simulink model "ofdm_txrx_supermimo_coded"  (Simulink 7.7) (continued)
== system ofdm_txrx_supermimo_coded/EDK Processor == (continued)
      | sm_TxRx_AutoReply_Match3_dout = xl_force(sm_TxRx_AutoReply_Match3, xlUnsigned, 0);
      | 
      | % sm_TxRx_AutoReply_Match2_dout = xfix({xlUnsigned, 32, 0}, 0);
      | sm_TxRx_AutoReply_Match2_dout = xl_force(sm_TxRx_AutoReply_Match2, xlUnsigned, 0);
      | 
      | % sm_TxRx_AutoReply_Action5_dout = xfix({xlUnsigned, 32, 0}, 0);
      | sm_TxRx_AutoReply_Action5_dout = xl_force(sm_TxRx_AutoReply_Action5, xlUnsigned, 0);
      | 
      | % sm_TxRx_AutoReply_Action4_dout = xfix({xlUnsigned, 32, 0}, 0);
      | sm_TxRx_AutoReply_Action4_dout = xl_force(sm_TxRx_AutoReply_Action4, xlUnsigned, 0);
      | 
      | % sm_TxRx_AutoReply_Match1_dout = xfix({xlUnsigned, 32, 0}, 0);
      | sm_TxRx_AutoReply_Match1_dout = xl_force(sm_TxRx_AutoReply_Match1, xlUnsigned, 0);
      | 
      | % sm_TxRx_AutoReply_Match0_dout = xfix({xlUnsigned, 32, 0}, 0);
      | sm_TxRx_AutoReply_Match0_dout = xl_force(sm_TxRx_AutoReply_Match0, xlUnsigned, 0);
      | 
      | 
      | % 'From FIFO' blocks
      | % 'To FIFO' blocks
      | % 'Shared Memory' blocks
      | % TxModulation_bus = xfix({xlUnsigned, 32, 0}, 0);
      | sm_TxModulation_bus = xl_force(sm_TxModulation, xlUnsigned, 0);
      | 
      | % TxHeaderTranslate_bus = xfix({xlUnsigned, 32, 0}, 0);
      | sm_TxHeaderTranslate_bus = xl_force(sm_TxHeaderTranslate, xlUnsigned, 0);
      | 
      | % ChannelEstimates_bus = xfix({xlUnsigned, 32, 0}, 0);
      | sm_ChannelEstimates_bus = xl_force(sm_ChannelEstimates, xlUnsigned, 0);
      | 
      | % RxModulation_bus = xfix({xlUnsigned, 32, 0}, 0);
      | sm_RxModulation_bus = xl_force(sm_RxModulation, xlUnsigned, 0);
      | 
      | % EVM_perSym_bus = xfix({xlUnsigned, 32, 0}, 0);
      | sm_EVM_perSym_bus = xl_force(sm_EVM_perSym, xlUnsigned, 0);
      | 
      | % EVM_perSC_bus = xfix({xlUnsigned, 32, 0}, 0);
      | sm_EVM_perSC_bus = xl_force(sm_EVM_perSC, xlUnsigned, 0);
      | 
      | % PktBufFreqOffsets_bus = xfix({xlUnsigned, 32, 0}, 0);
      | sm_PktBufFreqOffsets_bus = xl_force(sm_PktBufFreqOffsets, xlUnsigned, 0);
      | 
      | 
      | % 'dout' ports of 'From Register' blocks
      | 
      | % registered register mux output
      | persistent reg_bank_out_reg; reg_bank_out_reg = xl_state(0, {xlUnsigned, 32, 0});
      | reg_bank_out = reg_bank_out_reg;
      | 
      | if linearAddr == 41
      |     reg_bank_out_reg = sm_midPacketRSSI_bus;
      | elseif linearAddr == 42
      |     reg_bank_out_reg = sm_Tx_PktRunning_bus;
      | elseif linearAddr == 43
      |     reg_bank_out_reg = sm_Rx_pktDetEventCount_bus;
      | elseif linearAddr == 44
      |     reg_bank_out_reg = sm_Rx_coarseCFOest_bus;
      | elseif linearAddr == 45
      |     reg_bank_out_reg = sm_Rx_pilotCFOest_bus;
      | elseif linearAddr == 46
      |     reg_bank_out_reg = sm_Rx_pktDone_interruptStatus_bus;
      | elseif linearAddr == 47
      |     reg_bank_out_reg = sm_Rx_BER_TotalBits_bus;
      | elseif linearAddr == 48
      |     reg_bank_out_reg = sm_Rx_BER_Errors_bus;
      | elseif linearAddr == 49
      |     reg_bank_out_reg = sm_Rx_Gains_bus;
      | elseif linearAddr == 50
      |     reg_bank_out_reg = sm_pktDet_status_bus;
      | elseif linearAddr == 0
      |     reg_bank_out_reg = sm_FEC_Config_dout;
      | elseif linearAddr == 1
      |     reg_bank_out_reg = sm_Tx_OFDM_SymCounts_dout;
      | elseif linearAddr == 2
      |     reg_bank_out_reg = sm_Rx_OFDM_SymbolCounts_dout;
      | elseif linearAddr == 3
      |     reg_bank_out_reg = sm_TxRx_Pilots_Values_dout;
      | elseif linearAddr == 4
      |     reg_bank_out_reg = sm_TxRx_Pilots_Index_dout;
      | elseif linearAddr == 5
      |     reg_bank_out_reg = sm_TxRx_Interrupt_PktBuf_Ctrl_dout;
      | elseif linearAddr == 6
      |     reg_bank_out_reg = sm_TxRx_FFT_Scaling_dout;
      | elseif linearAddr == 7
      |     reg_bank_out_reg = sm_Tx_Start_Reset_Control_dout;
      | elseif linearAddr == 8
      |     reg_bank_out_reg = sm_Tx_ControlBits_dout;
      | elseif linearAddr == 9
      |     reg_bank_out_reg = sm_Tx_Delays_dout;
      | elseif linearAddr == 10
      |     reg_bank_out_reg = sm_Tx_Scaling_dout;
      | elseif linearAddr == 11
      |     reg_bank_out_reg = sm_pktDet_thresholds_dout;
      | elseif linearAddr == 12
      |     reg_bank_out_reg = sm_pktDet_durations_dout;
      | elseif linearAddr == 13
      |     reg_bank_out_reg = sm_pktDet_autoCorrParams_dout;
      | elseif linearAddr == 14
      |     reg_bank_out_reg = sm_pktDet_controlBits_dout;
      | elseif linearAddr == 15
      |     reg_bank_out_reg = sm_Rx_AF_TxScaling_dout;
      | elseif linearAddr == 16
      |     reg_bank_out_reg = sm_Rx_AF_Blanking_dout;
      | elseif linearAddr == 17
      |     reg_bank_out_reg = sm_Rx_PilotCalcParams_dout;
      | elseif linearAddr == 18
      |     reg_bank_out_reg = sm_Rx_Constellation_Scaling_dout;
      | elseif linearAddr == 19
      |     reg_bank_out_reg = sm_Rx_PktDet_Delay_dout;
      | elseif linearAddr == 20
      |     reg_bank_out_reg = sm_Rx_ChanEst_MinMag_dout;
      | elseif linearAddr == 21
      |     reg_bank_out_reg = sm_Rx_pktByteNums_dout;
      | elseif linearAddr == 22
      |     reg_bank_out_reg = sm_Rx_FixedPktLen_dout;
      | elseif linearAddr == 23
      |     reg_bank_out_reg = sm_Rx_PktDet_LongCorr_Thresholds_dout;
      | elseif linearAddr == 24
      |     reg_bank_out_reg = sm_Rx_PktDet_LongCorr_Params_dout;
      | elseif linearAddr == 25
      |     reg_bank_out_reg = sm_Rx_coarseCFO_correction_dout;
      | elseif linearAddr == 26
      |     reg_bank_out_reg = sm_Rx_PreCFO_PilotCalcCorrection_dout;
      | elseif linearAddr == 27
      |     reg_bank_out_reg = sm_Rx_PreCFO_Options_dout;
      | elseif linearAddr == 28
      |     reg_bank_out_reg = sm_Rx_ControlBits_dout;
      | elseif linearAddr == 29
      |     reg_bank_out_reg = sm_TxRx_AutoReply_Action3_dout;
      | elseif linearAddr == 30
      |     reg_bank_out_reg = sm_TxRx_AutoReply_Action2_dout;
      | elseif linearAddr == 31
      |     reg_bank_out_reg = sm_TxRx_AutoReply_Action1_dout;
      | elseif linearAddr == 32
      |     reg_bank_out_reg = sm_TxRx_AutoReply_Action0_dout;
      | elseif linearAddr == 33
      |     reg_bank_out_reg = sm_TxRx_AutoReply_Match5_dout;
      | elseif linearAddr == 34
      |     reg_bank_out_reg = sm_TxRx_AutoReply_Match4_dout;
      | elseif linearAddr == 35
      |     reg_bank_out_reg = sm_TxRx_AutoReply_Match3_dout;
      | elseif linearAddr == 36
      |     reg_bank_out_reg = sm_TxRx_AutoReply_Match2_dout;
      | elseif linearAddr == 37
      |     reg_bank_out_reg = sm_TxRx_AutoReply_Action5_dout;
      | elseif linearAddr == 38
      |     reg_bank_out_reg = sm_TxRx_AutoReply_Action4_dout;
      | elseif linearAddr == 39
      |     reg_bank_out_reg = sm_TxRx_AutoReply_Match1_dout;
      | elseif linearAddr == 40
      |     reg_bank_out_reg = sm_TxRx_AutoReply_Match0_dout;
      | 
      | end
      | 
      | 
      | % 'From FIFO' and 'To FIFO' blocks
      | 
      | 
      | 
      | 
      | 
      | opCode = xl_concat(addrAck, RNWReg, bankAddr, linearAddr);
      | 
      | % 'Shared Memory' blocks
      | 
      | sm_TxModulation_sel_value = xl_concat(xl_slice(linearAddr, ...
      |                                         xl_nbits(linearAddr) - 1, ...
      |                                         8) ...
      |                                     );
      | if sm_TxModulation_sel_value == xfix({xlUnsigned, ...
      |                                         xl_nbits(linearAddr) - 8, ...
      |                                         0}, ...
      |                                         4);
      |     sm_TxModulation_sel = true;
      | else
      |     sm_TxModulation_sel = false;
      | end
      | sm_TxHeaderTranslate_sel_value = xl_concat(xl_slice(linearAddr, ...
      |                                         xl_nbits(linearAddr) - 1, ...
      |                                         10) ...
      |                                     );
      | if sm_TxHeaderTranslate_sel_value == xfix({xlUnsigned, ...
      |                                         xl_nbits(linearAddr) - 10, ...
      |                                         0}, ...
      |                                         0);
      |     sm_TxHeaderTranslate_sel = true;
      | else
      |     sm_TxHeaderTranslate_sel = false;
      | end
      | sm_ChannelEstimates_sel_value = xl_concat(xl_slice(linearAddr, ...
      |                                         xl_nbits(linearAddr) - 1, ...
      |                                         8) ...
      |                                     );
      | if sm_ChannelEstimates_sel_value == xfix({xlUnsigned, ...
      |                                         xl_nbits(linearAddr) - 8, ...
      |                                         0}, ...
      |                                         5);
      |     sm_ChannelEstimates_sel = true;
      | else
      |     sm_ChannelEstimates_sel = false;
      | end
      | sm_RxModulation_sel_value = xl_concat(xl_slice(linearAddr, ...
      |                                         xl_nbits(linearAddr) - 1, ...
      |                                         8) ...
      |                                     );
      | if sm_RxModulation_sel_value == xfix({xlUnsigned, ...
      |                                         xl_nbits(linearAddr) - 8, ...
      |                                         0}, ...
      |                                         6);
      |     sm_RxModulation_sel = true;
      | else
      |     sm_RxModulation_sel = false;
      | end
      | sm_EVM_perSym_sel_value = xl_concat(xl_slice(linearAddr, ...
      |                                         xl_nbits(linearAddr) - 1, ...
      |                                         8) ...
      |                                     );
      | if sm_EVM_perSym_sel_value == xfix({xlUnsigned, ...
      |                                         xl_nbits(linearAddr) - 8, ...
      |                                         0}, ...
      |                                         7);
      |     sm_EVM_perSym_sel = true;
      | else
      |     sm_EVM_perSym_sel = false;
      | end
      | sm_EVM_perSC_sel_value = xl_concat(xl_slice(linearAddr, ...
      |                                         xl_nbits(linearAddr) - 1, ...
      |                                         6) ...
      |                                     );
      | if sm_EVM_perSC_sel_value == xfix({xlUnsigned, ...
      |                                         xl_nbits(linearAddr) - 6, ...
      |                                         0}, ...
      |                                         32);
      |     sm_EVM_perSC_sel = true;
      | else
      |     sm_EVM_perSC_sel = false;
      | end
      | sm_PktBufFreqOffsets_sel_value = xl_concat(xl_slice(linearAddr, ...
      |                                         xl_nbits(linearAddr) - 1, ...
      |                                         5) ...
      |                                     );
      | if sm_PktBufFreqOffsets_sel_value == xfix({xlUnsigned, ...
      |                                         xl_nbits(linearAddr) - 5, ...
      |                                         0}, ...
      |                                         66);
      |     sm_PktBufFreqOffsets_sel = true;
      | else
      |     sm_PktBufFreqOffsets_sel = false;
      | end
      | 
      | 
      | % registered Shared Memory mux output
      | persistent ram_bank_out_reg; ram_bank_out_reg = xl_state(0, {xlUnsigned, 32, 0});
      | ram_bank_out = ram_bank_out_reg;
      | if sm_TxModulation_sel
      |     ram_bank_out_reg = sm_TxModulation_bus;
      | elseif sm_TxHeaderTranslate_sel
      |     ram_bank_out_reg = sm_TxHeaderTranslate_bus;
      | elseif sm_ChannelEstimates_sel
      |     ram_bank_out_reg = sm_ChannelEstimates_bus;
      | elseif sm_RxModulation_sel
      |     ram_bank_out_reg = sm_RxModulation_bus;
      | elseif sm_EVM_perSym_sel
      |     ram_bank_out_reg = sm_EVM_perSym_bus;
      | elseif sm_EVM_perSC_sel
      |     ram_bank_out_reg = sm_EVM_perSC_bus;
      | elseif sm_PktBufFreqOffsets_sel
      |     ram_bank_out_reg = sm_PktBufFreqOffsets_bus;
      | end
      | 
      | % 'din' ports of 'Shared Memory' blocks
      | sm_TxModulation_din = xl_force(xl_slice(wrDBus, 4 - 1, 0), ...
      |                                  xlUnsigned, ...
      |                                  0);
      | sm_TxHeaderTranslate_din = xl_force(xl_slice(wrDBus, 10 - 1, 0), ...
      |                                  xlUnsigned, ...
      |                                  0);
      | sm_ChannelEstimates_din = xl_force(xl_slice(wrDBus, 32 - 1, 0), ...
      |                                  xlUnsigned, ...
      |                                  0);
      | sm_RxModulation_din = xl_force(xl_slice(wrDBus, 4 - 1, 0), ...
      |                                  xlUnsigned, ...
      |                                  0);
      | sm_EVM_perSym_din = xl_force(xl_slice(wrDBus, 32 - 1, 0), ...
      |                                  xlSigned, ...
      |                                  14);
      | sm_EVM_perSC_din = xl_force(xl_slice(wrDBus, 32 - 1, 0), ...
      |                                  xlSigned, ...
      |                                  22);
      | sm_PktBufFreqOffsets_din = xl_force(xl_slice(wrDBus, 32 - 1, 0), ...
      |                                  xlUnsigned, ...
      |                                  32);
      | 
      | 
      | % 'we' ports of 'Shared Memory' blocks
      | persistent sm_TxModulation_we_reg; sm_TxModulation_we_reg = xl_state(false, {xlBoolean});
      | sm_TxModulation_we = sm_TxModulation_we_reg;
      | opCode_sm_TxModulation = xl_concat(addrAck, ...
      |                                      RNWReg, ...
      |                                      bankAddr, ...
      |                                      xl_slice(linearAddr, ...
      |                                               xl_nbits(linearAddr) - 1, ...
      |                                               8) ...
      |                                     );
      | if opCode_sm_TxModulation == xl_concat(xfix({xlUnsigned, 4, 0}, 8), ...
      |                                          xfix({xlUnsigned, ...
      |                                                xl_nbits(linearAddr) - 8, ...
      |                                                0}, ...
      |                                                4) ...
      |                                         );
      |     sm_TxModulation_we_reg = true;
      | else
      |     sm_TxModulation_we_reg = false;
      | end
      | persistent sm_TxHeaderTranslate_we_reg; sm_TxHeaderTranslate_we_reg = xl_state(false, {xlBoolean});
      | sm_TxHeaderTranslate_we = sm_TxHeaderTranslate_we_reg;
      | opCode_sm_TxHeaderTranslate = xl_concat(addrAck, ...
      |                                      RNWReg, ...
      |                                      bankAddr, ...
      |                                      xl_slice(linearAddr, ...
      |                                               xl_nbits(linearAddr) - 1, ...
      |                                               10) ...
      |                                     );
      | if opCode_sm_TxHeaderTranslate == xl_concat(xfix({xlUnsigned, 4, 0}, 8), ...
      |                                          xfix({xlUnsigned, ...
      |                                                xl_nbits(linearAddr) - 10, ...
      |                                                0}, ...
      |                                                0) ...
      |                                         );
      |     sm_TxHeaderTranslate_we_reg = true;
      | else
      |     sm_TxHeaderTranslate_we_reg = false;
      | end
      | persistent sm_ChannelEstimates_we_reg; sm_ChannelEstimates_we_reg = xl_state(false, {xlBoolean});
      | sm_ChannelEstimates_we = sm_ChannelEstimates_we_reg;
      | opCode_sm_ChannelEstimates = xl_concat(addrAck, ...
      |                                      RNWReg, ...
      |                                      bankAddr, ...
      |                                      xl_slice(linearAddr, ...
      |                                               xl_nbits(linearAddr) - 1, ...
      |                                               8) ...
      |                                     );
      | if opCode_sm_ChannelEstimates == xl_concat(xfix({xlUnsigned, 4, 0}, 8), ...
      |                                          xfix({xlUnsigned, ...
      |                                                xl_nbits(linearAddr) - 8, ...
      |                                                0}, ...
      |                                                5) ...
      |                                         );
      |     sm_ChannelEstimates_we_reg = true;
      | else
      |     sm_ChannelEstimates_we_reg = false;
      | end
      | persistent sm_RxModulation_we_reg; sm_RxModulation_we_reg = xl_state(false, {xlBoolean});
      | sm_RxModulation_we = sm_RxModulation_we_reg;
      | opCode_sm_RxModulation = xl_concat(addrAck, ...
      |                                      RNWReg, ...
      |                                      bankAddr, ...
      |                                      xl_slice(linearAddr, ...
      |                                               xl_nbits(linearAddr) - 1, ...
      |                                               8) ...
      |                                     );
      | if opCode_sm_RxModulation == xl_concat(xfix({xlUnsigned, 4, 0}, 8), ...
      |                                          xfix({xlUnsigned, ...
      |                                                xl_nbits(linearAddr) - 8, ...
      |                                                0}, ...
      |                                                6) ...
      |                                         );
      |     sm_RxModulation_we_reg = true;
      | else
      |     sm_RxModulation_we_reg = false;
      | end
      | persistent sm_EVM_perSym_we_reg; sm_EVM_perSym_we_reg = xl_state(false, {xlBoolean});
      | sm_EVM_perSym_we = sm_EVM_perSym_we_reg;
      | opCode_sm_EVM_perSym = xl_concat(addrAck, ...
      |                                      RNWReg, ...
      |                                      bankAddr, ...
      |                                      xl_slice(linearAddr, ...
      |                                               xl_nbits(linearAddr) - 1, ...
      |                                               8) ...
      |                                     );
      | if opCode_sm_EVM_perSym == xl_concat(xfix({xlUnsigned, 4, 0}, 8), ...
      |                                          xfix({xlUnsigned, ...
      |                                                xl_nbits(linearAddr) - 8, ...
      |                                                0}, ...
      |                                                7) ...
      |                                         );
      |     sm_EVM_perSym_we_reg = true;
      | else
      |     sm_EVM_perSym_we_reg = false;
      | end
      | persistent sm_EVM_perSC_we_reg; sm_EVM_perSC_we_reg = xl_state(false, {xlBoolean});
      | sm_EVM_perSC_we = sm_EVM_perSC_we_reg;
      | opCode_sm_EVM_perSC = xl_concat(addrAck, ...
      |                                      RNWReg, ...
      |                                      bankAddr, ...
      |                                      xl_slice(linearAddr, ...
      |                                               xl_nbits(linearAddr) - 1, ...
      |                                               6) ...
      |                                     );
      | if opCode_sm_EVM_perSC == xl_concat(xfix({xlUnsigned, 4, 0}, 8), ...
      |                                          xfix({xlUnsigned, ...
      |                                                xl_nbits(linearAddr) - 6, ...
      |                                                0}, ...
      |                                                32) ...
      |                                         );
      |     sm_EVM_perSC_we_reg = true;
      | else
      |     sm_EVM_perSC_we_reg = false;
      | end
      | persistent sm_PktBufFreqOffsets_we_reg; sm_PktBufFreqOffsets_we_reg = xl_state(false, {xlBoolean});
      | sm_PktBufFreqOffsets_we = sm_PktBufFreqOffsets_we_reg;
      | opCode_sm_PktBufFreqOffsets = xl_concat(addrAck, ...
      |                                      RNWReg, ...
      |                                      bankAddr, ...
      |                                      xl_slice(linearAddr, ...
      |                                               xl_nbits(linearAddr) - 1, ...
      |                                               5) ...
      |                                     );
      | if opCode_sm_PktBufFreqOffsets == xl_concat(xfix({xlUnsigned, 4, 0}, 8), ...
      |                                          xfix({xlUnsigned, ...
      |                                                xl_nbits(linearAddr) - 5, ...
      |                                                0}, ...
      |                                                66) ...
      |                                         );
      |     sm_PktBufFreqOffsets_we_reg = true;
      | else
      |     sm_PktBufFreqOffsets_we_reg = false;
      | end
      | 
      | 
      | % 'addr' ports of 'Shared Memory' blocks
      | persistent sm_TxModulation_addr_reg; 
      | sm_TxModulation_addr_reg = xl_state(0, {xlUnsigned, 8, 0});
      | sm_TxModulation_addr = sm_TxModulation_addr_reg;
      | if addrAck == 1
      |     sm_TxModulation_addr_reg = xl_slice(linearAddr, 8, 0);
      | else
      |     sm_TxModulation_addr_reg = sm_TxModulation_addr_reg;
      | end
      | persistent sm_TxHeaderTranslate_addr_reg; 
      | sm_TxHeaderTranslate_addr_reg = xl_state(0, {xlUnsigned, 10, 0});
      | sm_TxHeaderTranslate_addr = sm_TxHeaderTranslate_addr_reg;
      | if addrAck == 1
      |     sm_TxHeaderTranslate_addr_reg = xl_slice(linearAddr, 10, 0);
      | else
      |     sm_TxHeaderTranslate_addr_reg = sm_TxHeaderTranslate_addr_reg;
      | end
      | persistent sm_ChannelEstimates_addr_reg; 
      | sm_ChannelEstimates_addr_reg = xl_state(0, {xlUnsigned, 8, 0});
      | sm_ChannelEstimates_addr = sm_ChannelEstimates_addr_reg;
      | if addrAck == 1
      |     sm_ChannelEstimates_addr_reg = xl_slice(linearAddr, 8, 0);
      | else
      |     sm_ChannelEstimates_addr_reg = sm_ChannelEstimates_addr_reg;
      | end
      | persistent sm_RxModulation_addr_reg; 
      | sm_RxModulation_addr_reg = xl_state(0, {xlUnsigned, 8, 0});
      | sm_RxModulation_addr = sm_RxModulation_addr_reg;
      | if addrAck == 1
      |     sm_RxModulation_addr_reg = xl_slice(linearAddr, 8, 0);
      | else
      |     sm_RxModulation_addr_reg = sm_RxModulation_addr_reg;
      | end
      | persistent sm_EVM_perSym_addr_reg; 
      | sm_EVM_perSym_addr_reg = xl_state(0, {xlUnsigned, 8, 0});
      | sm_EVM_perSym_addr = sm_EVM_perSym_addr_reg;
      | if addrAck == 1
      |     sm_EVM_perSym_addr_reg = xl_slice(linearAddr, 8, 0);
      | else
      |     sm_EVM_perSym_addr_reg = sm_EVM_perSym_addr_reg;
      | end
      | persistent sm_EVM_perSC_addr_reg; 
      | sm_EVM_perSC_addr_reg = xl_state(0, {xlUnsigned, 6, 0});
      | sm_EVM_perSC_addr = sm_EVM_perSC_addr_reg;
      | if addrAck == 1
      |     sm_EVM_perSC_addr_reg = xl_slice(linearAddr, 6, 0);
      | else
      |     sm_EVM_perSC_addr_reg = sm_EVM_perSC_addr_reg;
      | end
      | persistent sm_PktBufFreqOffsets_addr_reg; 
      | sm_PktBufFreqOffsets_addr_reg = xl_state(0, {xlUnsigned, 5, 0});
      | sm_PktBufFreqOffsets_addr = sm_PktBufFreqOffsets_addr_reg;
      | if addrAck == 1
      |     sm_PktBufFreqOffsets_addr_reg = xl_slice(linearAddr, 5, 0);
      | else
      |     sm_PktBufFreqOffsets_addr_reg = sm_PktBufFreqOffsets_addr_reg;
      | end
      | 
      | 
      | % 're' ports of 'From FIFO' blocks
      | 
      | 
      | % 'en' ports of 'To Register' blocks
      | if opCode == xl_concat(xfix({xlUnsigned, 4, 0}, 10), ...
      |                        xfix({xlUnsigned, xl_nbits(linearAddr), 0}, 0))
      |     sm_FEC_Config_en = true;
      | else
      |     sm_FEC_Config_en = false;
      | end
      | if opCode == xl_concat(xfix({xlUnsigned, 4, 0}, 10), ...
      |                        xfix({xlUnsigned, xl_nbits(linearAddr), 0}, 1))
      |     sm_Tx_OFDM_SymCounts_en = true;
      | else
      |     sm_Tx_OFDM_SymCounts_en = false;
      | end
      | if opCode == xl_concat(xfix({xlUnsigned, 4, 0}, 10), ...
      |                        xfix({xlUnsigned, xl_nbits(linearAddr), 0}, 2))
      |     sm_Rx_OFDM_SymbolCounts_en = true;
      | else
      |     sm_Rx_OFDM_SymbolCounts_en = false;
      | end
      | if opCode == xl_concat(xfix({xlUnsigned, 4, 0}, 10), ...
      |                        xfix({xlUnsigned, xl_nbits(linearAddr), 0}, 3))
      |     sm_TxRx_Pilots_Values_en = true;
      | else
      |     sm_TxRx_Pilots_Values_en = false;
      | end
      | if opCode == xl_concat(xfix({xlUnsigned, 4, 0}, 10), ...
      |                        xfix({xlUnsigned, xl_nbits(linearAddr), 0}, 4))
      |     sm_TxRx_Pilots_Index_en = true;
      | else
      |     sm_TxRx_Pilots_Index_en = false;
      | end
      | if opCode == xl_concat(xfix({xlUnsigned, 4, 0}, 10), ...
      |                        xfix({xlUnsigned, xl_nbits(linearAddr), 0}, 5))
      |     sm_TxRx_Interrupt_PktBuf_Ctrl_en = true;
      | else
      |     sm_TxRx_Interrupt_PktBuf_Ctrl_en = false;
      | end
      | if opCode == xl_concat(xfix({xlUnsigned, 4, 0}, 10), ...
      |                        xfix({xlUnsigned, xl_nbits(linearAddr), 0}, 6))
      |     sm_TxRx_FFT_Scaling_en = true;
      | else
      |     sm_TxRx_FFT_Scaling_en = false;
      | end
      | if opCode == xl_concat(xfix({xlUnsigned, 4, 0}, 10), ...
      |                        xfix({xlUnsigned, xl_nbits(linearAddr), 0}, 7))
      |     sm_Tx_Start_Reset_Control_en = true;
      | else
      |     sm_Tx_Start_Reset_Control_en = false;
      | end
      | if opCode == xl_concat(xfix({xlUnsigned, 4, 0}, 10), ...
      |                        xfix({xlUnsigned, xl_nbits(linearAddr), 0}, 8))
      |     sm_Tx_ControlBits_en = true;
      | else
      |     sm_Tx_ControlBits_en = false;
      | end
      | if opCode == xl_concat(xfix({xlUnsigned, 4, 0}, 10), ...
      |                        xfix({xlUnsigned, xl_nbits(linearAddr), 0}, 9))
      |     sm_Tx_Delays_en = true;
      | else
      |     sm_Tx_Delays_en = false;
      | end
      | if opCode == xl_concat(xfix({xlUnsigned, 4, 0}, 10), ...
      |                        xfix({xlUnsigned, xl_nbits(linearAddr), 0}, 10))
      |     sm_Tx_Scaling_en = true;
      | else
      |     sm_Tx_Scaling_en = false;
      | end
      | if opCode == xl_concat(xfix({xlUnsigned, 4, 0}, 10), ...
      |                        xfix({xlUnsigned, xl_nbits(linearAddr), 0}, 11))
      |     sm_pktDet_thresholds_en = true;
      | else
      |     sm_pktDet_thresholds_en = false;
      | end
      | if opCode == xl_concat(xfix({xlUnsigned, 4, 0}, 10), ...
      |                        xfix({xlUnsigned, xl_nbits(linearAddr), 0}, 12))
      |     sm_pktDet_durations_en = true;
      | else
      |     sm_pktDet_durations_en = false;
      | end
      | if opCode == xl_concat(xfix({xlUnsigned, 4, 0}, 10), ...
      |                        xfix({xlUnsigned, xl_nbits(linearAddr), 0}, 13))
      |     sm_pktDet_autoCorrParams_en = true;
      | else
      |     sm_pktDet_autoCorrParams_en = false;
      | end
      | if opCode == xl_concat(xfix({xlUnsigned, 4, 0}, 10), ...
      |                        xfix({xlUnsigned, xl_nbits(linearAddr), 0}, 14))
      |     sm_pktDet_controlBits_en = true;
      | else
      |     sm_pktDet_controlBits_en = false;
      | end
      | if opCode == xl_concat(xfix({xlUnsigned, 4, 0}, 10), ...
      |                        xfix({xlUnsigned, xl_nbits(linearAddr), 0}, 15))
      |     sm_Rx_AF_TxScaling_en = true;
      | else
      |     sm_Rx_AF_TxScaling_en = false;
      | end
      | if opCode == xl_concat(xfix({xlUnsigned, 4, 0}, 10), ...
      |                        xfix({xlUnsigned, xl_nbits(linearAddr), 0}, 16))
      |     sm_Rx_AF_Blanking_en = true;
      | else
      |     sm_Rx_AF_Blanking_en = false;
      | end
      | if opCode == xl_concat(xfix({xlUnsigned, 4, 0}, 10), ...
      |                        xfix({xlUnsigned, xl_nbits(linearAddr), 0}, 17))
      |     sm_Rx_PilotCalcParams_en = true;
      | else
      |     sm_Rx_PilotCalcParams_en = false;
      | end
      | if opCode == xl_concat(xfix({xlUnsigned, 4, 0}, 10), ...
      |                        xfix({xlUnsigned, xl_nbits(linearAddr), 0}, 18))
      |     sm_Rx_Constellation_Scaling_en = true;
      | else
      |     sm_Rx_Constellation_Scaling_en = false;
      | end
      | if opCode == xl_concat(xfix({xlUnsigned, 4, 0}, 10), ...
      |                        xfix({xlUnsigned, xl_nbits(linearAddr), 0}, 19))
      |     sm_Rx_PktDet_Delay_en = true;
      | else
      |     sm_Rx_PktDet_Delay_en = false;
      | end
      | if opCode == xl_concat(xfix({xlUnsigned, 4, 0}, 10), ...
      |                        xfix({xlUnsigned, xl_nbits(linearAddr), 0}, 20))
      |     sm_Rx_ChanEst_MinMag_en = true;
      | else
      |     sm_Rx_ChanEst_MinMag_en = false;
      | end
      | if opCode == xl_concat(xfix({xlUnsigned, 4, 0}, 10), ...
      |                        xfix({xlUnsigned, xl_nbits(linearAddr), 0}, 21))
      |     sm_Rx_pktByteNums_en = true;
      | else
      |     sm_Rx_pktByteNums_en = false;
      | end
      | if opCode == xl_concat(xfix({xlUnsigned, 4, 0}, 10), ...
      |                        xfix({xlUnsigned, xl_nbits(linearAddr), 0}, 22))
      |     sm_Rx_FixedPktLen_en = true;
      | else
      |     sm_Rx_FixedPktLen_en = false;
      | end
      | if opCode == xl_concat(xfix({xlUnsigned, 4, 0}, 10), ...
      |                        xfix({xlUnsigned, xl_nbits(linearAddr), 0}, 23))
      |     sm_Rx_PktDet_LongCorr_Thresholds_en = true;
      | else
      |     sm_Rx_PktDet_LongCorr_Thresholds_en = false;
      | end
      | if opCode == xl_concat(xfix({xlUnsigned, 4, 0}, 10), ...
      |                        xfix({xlUnsigned, xl_nbits(linearAddr), 0}, 24))
      |     sm_Rx_PktDet_LongCorr_Params_en = true;
      | else
      |     sm_Rx_PktDet_LongCorr_Params_en = false;
      | end
      | if opCode == xl_concat(xfix({xlUnsigned, 4, 0}, 10), ...
      |                        xfix({xlUnsigned, xl_nbits(linearAddr), 0}, 25))
      |     sm_Rx_coarseCFO_correction_en = true;
      | else
      |     sm_Rx_coarseCFO_correction_en = false;
      | end
      | if opCode == xl_concat(xfix({xlUnsigned, 4, 0}, 10), ...
      |                        xfix({xlUnsigned, xl_nbits(linearAddr), 0}, 26))
      |     sm_Rx_PreCFO_PilotCalcCorrection_en = true;
      | else
      |     sm_Rx_PreCFO_PilotCalcCorrection_en = false;
      | end
      | if opCode == xl_concat(xfix({xlUnsigned, 4, 0}, 10), ...
      |                        xfix({xlUnsigned, xl_nbits(linearAddr), 0}, 27))
      |     sm_Rx_PreCFO_Options_en = true;
      | else
      |     sm_Rx_PreCFO_Options_en = false;
      | end
      | if opCode == xl_concat(xfix({xlUnsigned, 4, 0}, 10), ...
      |                        xfix({xlUnsigned, xl_nbits(linearAddr), 0}, 28))
      |     sm_Rx_ControlBits_en = true;
      | else
      |     sm_Rx_ControlBits_en = false;
      | end
      | if opCode == xl_concat(xfix({xlUnsigned, 4, 0}, 10), ...
      |                        xfix({xlUnsigned, xl_nbits(linearAddr), 0}, 29))
      |     sm_TxRx_AutoReply_Action3_en = true;
      | else
      |     sm_TxRx_AutoReply_Action3_en = false;
      | end
      | if opCode == xl_concat(xfix({xlUnsigned, 4, 0}, 10), ...
      |                        xfix({xlUnsigned, xl_nbits(linearAddr), 0}, 30))
      |     sm_TxRx_AutoReply_Action2_en = true;
      | else
      |     sm_TxRx_AutoReply_Action2_en = false;
      | end
      | if opCode == xl_concat(xfix({xlUnsigned, 4, 0}, 10), ...
      |                        xfix({xlUnsigned, xl_nbits(linearAddr), 0}, 31))
      |     sm_TxRx_AutoReply_Action1_en = true;
      | else
      |     sm_TxRx_AutoReply_Action1_en = false;
      | end
      | if opCode == xl_concat(xfix({xlUnsigned, 4, 0}, 10), ...
      |                        xfix({xlUnsigned, xl_nbits(linearAddr), 0}, 32))
      |     sm_TxRx_AutoReply_Action0_en = true;
      | else
      |     sm_TxRx_AutoReply_Action0_en = false;
      | end
      | if opCode == xl_concat(xfix({xlUnsigned, 4, 0}, 10), ...
      |                        xfix({xlUnsigned, xl_nbits(linearAddr), 0}, 33))
      |     sm_TxRx_AutoReply_Match5_en = true;
      | else
      |     sm_TxRx_AutoReply_Match5_en = false;
      | end
      | if opCode == xl_concat(xfix({xlUnsigned, 4, 0}, 10), ...
      |                        xfix({xlUnsigned, xl_nbits(linearAddr), 0}, 34))
      |     sm_TxRx_AutoReply_Match4_en = true;
      | else
      |     sm_TxRx_AutoReply_Match4_en = false;
      | end
      | if opCode == xl_concat(xfix({xlUnsigned, 4, 0}, 10), ...
      |                        xfix({xlUnsigned, xl_nbits(linearAddr), 0}, 35))
      |     sm_TxRx_AutoReply_Match3_en = true;
      | else
      |     sm_TxRx_AutoReply_Match3_en = false;
      | end
      | if opCode == xl_concat(xfix({xlUnsigned, 4, 0}, 10), ...
      |                        xfix({xlUnsigned, xl_nbits(linearAddr), 0}, 36))
      |     sm_TxRx_AutoReply_Match2_en = true;
      | else
      |     sm_TxRx_AutoReply_Match2_en = false;
      | end
      | if opCode == xl_concat(xfix({xlUnsigned, 4, 0}, 10), ...
      |                        xfix({xlUnsigned, xl_nbits(linearAddr), 0}, 37))
      |     sm_TxRx_AutoReply_Action5_en = true;
      | else
      |     sm_TxRx_AutoReply_Action5_en = false;
      | end
      | if opCode == xl_concat(xfix({xlUnsigned, 4, 0}, 10), ...
      |                        xfix({xlUnsigned, xl_nbits(linearAddr), 0}, 38))
      |     sm_TxRx_AutoReply_Action4_en = true;
      | else
      |     sm_TxRx_AutoReply_Action4_en = false;
      | end
      | if opCode == xl_concat(xfix({xlUnsigned, 4, 0}, 10), ...
      |                        xfix({xlUnsigned, xl_nbits(linearAddr), 0}, 39))
      |     sm_TxRx_AutoReply_Match1_en = true;
      | else
      |     sm_TxRx_AutoReply_Match1_en = false;
      | end
      | if opCode == xl_concat(xfix({xlUnsigned, 4, 0}, 10), ...
      |                        xfix({xlUnsigned, xl_nbits(linearAddr), 0}, 40))
      |     sm_TxRx_AutoReply_Match0_en = true;
      | else
      |     sm_TxRx_AutoReply_Match0_en = false;
      | end
      | 
      | 
      | % 'din' ports of 'To FIFO' blocks
      | 
      | 
      | % 'we' ports of 'To FIFO' blocks
      | 
      | 
      | % 'din' ports of 'To Register' blocks
      | sm_FEC_Config_din = xl_force(xl_slice(wrDBus, 32 - 1, 0), ...
      |                                  xlUnsigned, ...
      |                                  0);
      | sm_Tx_OFDM_SymCounts_din = xl_force(xl_slice(wrDBus, 32 - 1, 0), ...
      |                                  xlUnsigned, ...
      |                                  0);
      | sm_Rx_OFDM_SymbolCounts_din = xl_force(xl_slice(wrDBus, 32 - 1, 0), ...
      |                                  xlUnsigned, ...
      |                                  0);
      | sm_TxRx_Pilots_Values_din = xl_force(xl_slice(wrDBus, 32 - 1, 0), ...
      |                                  xlUnsigned, ...
      |                                  0);
      | sm_TxRx_Pilots_Index_din = xl_force(xl_slice(wrDBus, 32 - 1, 0), ...
      |                                  xlUnsigned, ...
      |                                  0);
      | sm_TxRx_Interrupt_PktBuf_Ctrl_din = xl_force(xl_slice(wrDBus, 32 - 1, 0), ...
      |                                  xlUnsigned, ...
      |                                  0);
      | sm_TxRx_FFT_Scaling_din = xl_force(xl_slice(wrDBus, 12 - 1, 0), ...
      |                                  xlUnsigned, ...
      |                                  0);
      | sm_Tx_Start_Reset_Control_din = xl_force(xl_slice(wrDBus, 32 - 1, 0), ...
      |                                  xlUnsigned, ...
      |                                  0);
      | sm_Tx_ControlBits_din = xl_force(xl_slice(wrDBus, 32 - 1, 0), ...
      |                                  xlUnsigned, ...
      |                                  0);
      | sm_Tx_Delays_din = xl_force(xl_slice(wrDBus, 32 - 1, 0), ...
      |                                  xlUnsigned, ...
      |                                  0);
      | sm_Tx_Scaling_din = xl_force(xl_slice(wrDBus, 32 - 1, 0), ...
      |                                  xlUnsigned, ...
      |                                  0);
      | sm_pktDet_thresholds_din = xl_force(xl_slice(wrDBus, 32 - 1, 0), ...
      |                                  xlUnsigned, ...
      |                                  0);
      | sm_pktDet_durations_din = xl_force(xl_slice(wrDBus, 32 - 1, 0), ...
      |                                  xlUnsigned, ...
      |                                  0);
      | sm_pktDet_autoCorrParams_din = xl_force(xl_slice(wrDBus, 32 - 1, 0), ...
      |                                  xlUnsigned, ...
      |                                  0);
      | sm_pktDet_controlBits_din = xl_force(xl_slice(wrDBus, 32 - 1, 0), ...
      |                                  xlUnsigned, ...
      |                                  0);
      | sm_Rx_AF_TxScaling_din = xl_force(xl_slice(wrDBus, 32 - 1, 0), ...
      |                                  xlUnsigned, ...
      |                                  0);
      | sm_Rx_AF_Blanking_din = xl_force(xl_slice(wrDBus, 32 - 1, 0), ...
      |                                  xlUnsigned, ...
      |                                  0);
      | sm_Rx_PilotCalcParams_din = xl_force(xl_slice(wrDBus, 32 - 1, 0), ...
      |                                  xlUnsigned, ...
      |                                  0);
      | sm_Rx_Constellation_Scaling_din = xl_force(xl_slice(wrDBus, 32 - 1, 0), ...
      |                                  xlUnsigned, ...
      |                                  0);
      | sm_Rx_PktDet_Delay_din = xl_force(xl_slice(wrDBus, 32 - 1, 0), ...
      |                                  xlUnsigned, ...
      |                                  0);
      | sm_Rx_ChanEst_MinMag_din = xl_force(xl_slice(wrDBus, 32 - 1, 0), ...
      |                                  xlUnsigned, ...
      |                                  0);
      | sm_Rx_pktByteNums_din = xl_force(xl_slice(wrDBus, 32 - 1, 0), ...
      |                                  xlUnsigned, ...
      |                                  0);
      | sm_Rx_FixedPktLen_din = xl_force(xl_slice(wrDBus, 32 - 1, 0), ...
      |                                  xlUnsigned, ...
      |                                  0);
      | sm_Rx_PktDet_LongCorr_Thresholds_din = xl_force(xl_slice(wrDBus, 32 - 1, 0), ...
      |                                  xlUnsigned, ...
      |                                  0);
      | sm_Rx_PktDet_LongCorr_Params_din = xl_force(xl_slice(wrDBus, 32 - 1, 0), ...
      |                                  xlUnsigned, ...
      |                                  0);
      | sm_Rx_coarseCFO_correction_din = xl_force(xl_slice(wrDBus, 32 - 1, 0), ...
      |                                  xlUnsigned, ...
      |                                  0);
      | sm_Rx_PreCFO_PilotCalcCorrection_din = xl_force(xl_slice(wrDBus, 32 - 1, 0), ...
      |                                  xlUnsigned, ...
      |                                  0);
      | sm_Rx_PreCFO_Options_din = xl_force(xl_slice(wrDBus, 32 - 1, 0), ...
      |                                  xlUnsigned, ...
      |                                  0);
      | sm_Rx_ControlBits_din = xl_force(xl_slice(wrDBus, 32 - 1, 0), ...
      |                                  xlUnsigned, ...
      |                                  0);
      | sm_TxRx_AutoReply_Action3_din = xl_force(xl_slice(wrDBus, 32 - 1, 0), ...
      |                                  xlUnsigned, ...
      |                                  0);
      | sm_TxRx_AutoReply_Action2_din = xl_force(xl_slice(wrDBus, 32 - 1, 0), ...
      |                                  xlUnsigned, ...
      |                                  0);
      | sm_TxRx_AutoReply_Action1_din = xl_force(xl_slice(wrDBus, 32 - 1, 0), ...
      |                                  xlUnsigned, ...
      |                                  0);
      | sm_TxRx_AutoReply_Action0_din = xl_force(xl_slice(wrDBus, 32 - 1, 0), ...
      |                                  xlUnsigned, ...
      |                                  0);
      | sm_TxRx_AutoReply_Match5_din = xl_force(xl_slice(wrDBus, 32 - 1, 0), ...
      |                                  xlUnsigned, ...
      |                                  0);
      | sm_TxRx_AutoReply_Match4_din = xl_force(xl_slice(wrDBus, 32 - 1, 0), ...
      |                                  xlUnsigned, ...
      |                                  0);
      | sm_TxRx_AutoReply_Match3_din = xl_force(xl_slice(wrDBus, 32 - 1, 0), ...
      |                                  xlUnsigned, ...
      |                                  0);
      | sm_TxRx_AutoReply_Match2_din = xl_force(xl_slice(wrDBus, 32 - 1, 0), ...
      |                                  xlUnsigned, ...
      |                                  0);
      | sm_TxRx_AutoReply_Action5_din = xl_force(xl_slice(wrDBus, 32 - 1, 0), ...
      |                                  xlUnsigned, ...
      |                                  0);
      | sm_TxRx_AutoReply_Action4_din = xl_force(xl_slice(wrDBus, 32 - 1, 0), ...
      |                                  xlUnsigned, ...
      |                                  0);
      | sm_TxRx_AutoReply_Match1_din = xl_force(xl_slice(wrDBus, 32 - 1, 0), ...
      |                                  xlUnsigned, ...
      |                                  0);
      | sm_TxRx_AutoReply_Match0_din = xl_force(xl_slice(wrDBus, 32 - 1, 0), ...
      |                                  xlUnsigned, ...
      |                                  0);
      | 
      | 
      | persistent read_bank_out_reg; read_bank_out_reg = xl_state(0, {xlUnsigned, 32, 0});
      | read_bank_out = read_bank_out_reg;
      | 
      | persistent bankAddr_reg; bankAddr_reg = xl_state(0, bankAddr);
      | 
      | if bankAddr_reg == 0
      |     % Bank 0: Shared Memories
      |     read_bank_out_reg = ram_bank_out;
      | elseif bankAddr_reg == 1
      |     % Bank 1: From/To FIFOs
      |     read_bank_out_reg =  0;
      | elseif bankAddr_reg == 2
      |     % Bank 2: From/To Registers
      |     read_bank_out_reg = reg_bank_out;
      | elseif bankAddr_reg == 3
      |     % Bank 3: Configuration Registers
      |     read_bank_out_reg = 0;
      | end
      | 
      | bankAddr_reg = bankAddr;
  sg_plb_addrpref : xbsIndex_r4/Gateway In  gui_display_data_type=Fixed-point  arith_type=Unsigned  n_bits=16  bin_pt=0  preci_type=Single  exp_width=8  frac_width=24  quantization="Round  (unbiased: +/- Inf)"  overflow=Saturate  period=xlGetNormalizedPeriod()  dbl_ovrd=off  timing_constraint=None  locs_specified=off  LOCs={}  inherit_from_input=off  UseAsADC=off  ADCChannel='1'  hdl_port=on  block_type=gatewayin
      settings: infoedit="Gateway in block.  Converts inputs of type Simulink integer, single, double and fixed point to  Xilinx fixed-point or floating-point data type.<br><br>Hardware notes:  In hardware these blocks become top level input ports."  sginterface={'Xilinx'=>{'jtaghwcosim'=>{'non_memory_mapped_port'=>'sg_plb_addrpref'}},'iopad'=>{'constraint'=>'#'}}
  wires:
  plb_decode/2 -> plb_memmap/5, Sl_addrAck/1  "Sl_addrAck"
  plb_decode/4 -> Sl_wrDAck/1, Sl_wrComp/1  "Sl_wrDAck"
  plb_memmap/104 -> Shared Memory6/3  "PktBufFreqOffsets_we"
  plb_memmap/103 -> Shared Memory6/2  "PktBufFreqOffsets_din"
  plb_memmap/102 -> Shared Memory6/1  "PktBufFreqOffsets_addr"
  plb_memmap/101 -> Shared Memory5/3  "EVM_perSC_we"
  plb_memmap/100 -> Shared Memory5/2  "EVM_perSC_din"
  plb_memmap/99 -> Shared Memory5/1  "EVM_perSC_addr"
  plb_memmap/98 -> Shared Memory4/3  "EVM_perSym_we"
  plb_memmap/97 -> Shared Memory4/2  "EVM_perSym_din"
  plb_memmap/96 -> Shared Memory4/1  "EVM_perSym_addr"
  plb_memmap/95 -> Shared Memory3/3  "RxModulation_we"
  plb_memmap/94 -> Shared Memory3/2  "RxModulation_din"
  plb_memmap/93 -> Shared Memory3/1  "RxModulation_addr"
  plb_memmap/92 -> Shared Memory2/3  "ChannelEstimates_we"
  plb_memmap/91 -> Shared Memory2/2  "ChannelEstimates_din"
  plb_memmap/90 -> Shared Memory2/1  "ChannelEstimates_addr"
  plb_memmap/89 -> Shared Memory1/3  "TxHeaderTranslate_we"
  plb_memmap/88 -> Shared Memory1/2  "TxHeaderTranslate_din"
  plb_memmap/87 -> Shared Memory1/1  "TxHeaderTranslate_addr"
  plb_memmap/86 -> Shared Memory/3  "TxModulation_we"
  plb_memmap/85 -> Shared Memory/2  "TxModulation_din"
  plb_memmap/84 -> Shared Memory/1  "TxModulation_addr"
  plb_memmap/83 -> To Register40/2  "TxRx_AutoReply_Match0_en"
  plb_memmap/82 -> To Register40/1  "TxRx_AutoReply_Match0_din"
  plb_memmap/81 -> To Register39/2  "TxRx_AutoReply_Match1_en"
  plb_memmap/80 -> To Register39/1  "TxRx_AutoReply_Match1_din"
  plb_memmap/79 -> To Register38/2  "TxRx_AutoReply_Action4_en"
  plb_memmap/78 -> To Register38/1  "TxRx_AutoReply_Action4_din"
  plb_memmap/77 -> To Register37/2  "TxRx_AutoReply_Action5_en"
  plb_memmap/76 -> To Register37/1  "TxRx_AutoReply_Action5_din"
  plb_memmap/75 -> To Register36/2  "TxRx_AutoReply_Match2_en"
  plb_memmap/74 -> To Register36/1  "TxRx_AutoReply_Match2_din"
  plb_memmap/73 -> To Register35/2  "TxRx_AutoReply_Match3_en"
  plb_memmap/72 -> To Register35/1  "TxRx_AutoReply_Match3_din"
  plb_memmap/71 -> To Register34/2  "TxRx_AutoReply_Match4_en"
  plb_memmap/70 -> To Register34/1  "TxRx_AutoReply_Match4_din"
  plb_memmap/69 -> To Register33/2  "TxRx_AutoReply_Match5_en"
  plb_memmap/68 -> To Register33/1  "TxRx_AutoReply_Match5_din"
  plb_memmap/67 -> To Register32/2  "TxRx_AutoReply_Action0_en"
  plb_memmap/66 -> To Register32/1  "TxRx_AutoReply_Action0_din"
  plb_memmap/65 -> To Register31/2  "TxRx_AutoReply_Action1_en"
  plb_memmap/64 -> To Register31/1  "TxRx_AutoReply_Action1_din"
  plb_memmap/63 -> To Register30/2  "TxRx_AutoReply_Action2_en"
  plb_memmap/62 -> To Register30/1  "TxRx_AutoReply_Action2_din"
  plb_memmap/61 -> To Register29/2  "TxRx_AutoReply_Action3_en"
  plb_memmap/60 -> To Register29/1  "TxRx_AutoReply_Action3_din"
  plb_memmap/59 -> To Register28/2  "Rx_ControlBits_en"
  plb_memmap/58 -> To Register28/1  "Rx_ControlBits_din"
  plb_memmap/57 -> To Register27/2  "Rx_PreCFO_Options_en"
  plb_memmap/56 -> To Register27/1  "Rx_PreCFO_Options_din"
  plb_memmap/55 -> To Register26/2  "Rx_PreCFO_PilotCalcCorrection_en"
  plb_memmap/54 -> To Register26/1  "Rx_PreCFO_PilotCalcCorrection_din"
  plb_memmap/53 -> To Register25/2  "Rx_coarseCFO_correction_en"
  plb_memmap/52 -> To Register25/1  "Rx_coarseCFO_correction_din"
  plb_memmap/51 -> To Register24/2  "Rx_PktDet_LongCorr_Params_en"
  plb_memmap/50 -> To Register24/1  "Rx_PktDet_LongCorr_Params_din"
  plb_memmap/49 -> To Register23/2  "Rx_PktDet_LongCorr_Thresholds_en"
  plb_memmap/48 -> To Register23/1  "Rx_PktDet_LongCorr_Thresholds_din"
  plb_memmap/47 -> To Register22/2  "Rx_FixedPktLen_en"
  plb_memmap/46 -> To Register22/1  "Rx_FixedPktLen_din"
  plb_memmap/45 -> To Register21/2  "Rx_pktByteNums_en"
  plb_memmap/44 -> To Register21/1  "Rx_pktByteNums_din"
  plb_memmap/43 -> To Register20/2  "Rx_ChanEst_MinMag_en"
  plb_memmap/42 -> To Register20/1  "Rx_ChanEst_MinMag_din"
  plb_memmap/41 -> To Register19/2  "Rx_PktDet_Delay_en"
  plb_memmap/40 -> To Register19/1  "Rx_PktDet_Delay_din"
  plb_memmap/39 -> To Register18/2  "Rx_Constellation_Scaling_en"
  plb_memmap/38 -> To Register18/1  "Rx_Constellation_Scaling_din"
  plb_memmap/37 -> To Register17/2  "Rx_PilotCalcParams_en"
  plb_memmap/36 -> To Register17/1  "Rx_PilotCalcParams_din"
  plb_memmap/35 -> To Register16/2  "Rx_AF_Blanking_en"
  plb_memmap/34 -> To Register16/1  "Rx_AF_Blanking_din"
  plb_memmap/33 -> To Register15/2  "Rx_AF_TxScaling_en"
  plb_memmap/32 -> To Register15/1  "Rx_AF_TxScaling_din"
  plb_memmap/31 -> To Register14/2  "pktDet_controlBits_en"
  plb_memmap/30 -> To Register14/1  "pktDet_controlBits_din"
  plb_memmap/29 -> To Register13/2  "pktDet_autoCorrParams_en"
  plb_memmap/28 -> To Register13/1  "pktDet_autoCorrParams_din"
  plb_memmap/27 -> To Register12/2  "pktDet_durations_en"
  plb_memmap/26 -> To Register12/1  "pktDet_durations_din"
  plb_memmap/25 -> To Register11/2  "pktDet_thresholds_en"
  plb_memmap/24 -> To Register11/1  "pktDet_thresholds_din"
  plb_memmap/23 -> To Register10/2  "Tx_Scaling_en"
  plb_memmap/22 -> To Register10/1  "Tx_Scaling_din"
  plb_memmap/21 -> To Register9/2  "Tx_Delays_en"
  plb_memmap/20 -> To Register9/1  "Tx_Delays_din"
  plb_memmap/19 -> To Register8/2  "Tx_ControlBits_en"
  plb_memmap/18 -> To Register8/1  "Tx_ControlBits_din"
  plb_memmap/17 -> To Register7/2  "Tx_Start_Reset_Control_en"
  plb_memmap/16 -> To Register7/1  "Tx_Start_Reset_Control_din"
  plb_memmap/15 -> To Register6/2  "TxRx_FFT_Scaling_en"
  plb_memmap/14 -> To Register6/1  "TxRx_FFT_Scaling_din"
  plb_memmap/13 -> To Register5/2  "TxRx_Interrupt_PktBuf_Ctrl_en"
  plb_memmap/12 -> To Register5/1  "TxRx_Interrupt_PktBuf_Ctrl_din"
  plb_memmap/11 -> To Register4/2  "TxRx_Pilots_Index_en"
  plb_memmap/10 -> To Register4/1  "TxRx_Pilots_Index_din"
  plb_memmap/9 -> To Register3/2  "TxRx_Pilots_Values_en"
  plb_memmap/8 -> To Register3/1  "TxRx_Pilots_Values_din"
  plb_memmap/7 -> To Register2/2  "Rx_OFDM_SymbolCounts_en"
  plb_memmap/6 -> To Register2/1  "Rx_OFDM_SymbolCounts_din"
  plb_memmap/5 -> To Register1/2  "Tx_OFDM_SymCounts_en"
  plb_memmap/4 -> To Register1/1  "Tx_OFDM_SymCounts_din"
  plb_memmap/3 -> To Register/2  "FEC_Config_en"
  plb_memmap/2 -> To Register/1  "FEC_Config_din"
  plb_memmap/1 -> plb_decode/6  "rdData"
  Shared Memory6/1 -> plb_memmap/63  "PktBufFreqOffsets_dout"
  Shared Memory5/1 -> plb_memmap/62  "EVM_perSC_dout"
  Shared Memory4/1 -> plb_memmap/61  "EVM_perSym_dout"
  Shared Memory3/1 -> plb_memmap/60  "RxModulation_dout"
  Shared Memory2/1 -> plb_memmap/59  "ChannelEstimates_dout"
  Shared Memory1/1 -> plb_memmap/58  "TxHeaderTranslate_dout"
  Shared Memory/1 -> plb_memmap/57  "TxModulation_dout"
  To Register40/1 -> plb_memmap/56  "TxRx_AutoReply_Match0_dout"
  To Register39/1 -> plb_memmap/55  "TxRx_AutoReply_Match1_dout"
  To Register38/1 -> plb_memmap/54  "TxRx_AutoReply_Action4_dout"
  To Register37/1 -> plb_memmap/53  "TxRx_AutoReply_Action5_dout"
  To Register36/1 -> plb_memmap/52  "TxRx_AutoReply_Match2_dout"
  To Register35/1 -> plb_memmap/51  "TxRx_AutoReply_Match3_dout"
  To Register34/1 -> plb_memmap/50  "TxRx_AutoReply_Match4_dout"
  To Register33/1 -> plb_memmap/49  "TxRx_AutoReply_Match5_dout"
  To Register32/1 -> plb_memmap/48  "TxRx_AutoReply_Action0_dout"
  To Register31/1 -> plb_memmap/47  "TxRx_AutoReply_Action1_dout"
  To Register30/1 -> plb_memmap/46  "TxRx_AutoReply_Action2_dout"
  To Register29/1 -> plb_memmap/45  "TxRx_AutoReply_Action3_dout"
  To Register28/1 -> plb_memmap/44  "Rx_ControlBits_dout"
  To Register27/1 -> plb_memmap/43  "Rx_PreCFO_Options_dout"
  To Register26/1 -> plb_memmap/42  "Rx_PreCFO_PilotCalcCorrection_dout"
  To Register25/1 -> plb_memmap/41  "Rx_coarseCFO_correction_dout"
  To Register24/1 -> plb_memmap/40  "Rx_PktDet_LongCorr_Params_dout"
  To Register23/1 -> plb_memmap/39  "Rx_PktDet_LongCorr_Thresholds_dout"
  To Register22/1 -> plb_memmap/38  "Rx_FixedPktLen_dout"
  To Register21/1 -> plb_memmap/37  "Rx_pktByteNums_dout"
  To Register20/1 -> plb_memmap/36  "Rx_ChanEst_MinMag_dout"
  To Register19/1 -> plb_memmap/35  "Rx_PktDet_Delay_dout"
  To Register18/1 -> plb_memmap/34  "Rx_Constellation_Scaling_dout"
  To Register17/1 -> plb_memmap/33  "Rx_PilotCalcParams_dout"
  To Register16/1 -> plb_memmap/32  "Rx_AF_Blanking_dout"
  To Register15/1 -> plb_memmap/31  "Rx_AF_TxScaling_dout"
  To Register14/1 -> plb_memmap/30  "pktDet_controlBits_dout"
  To Register13/1 -> plb_memmap/29  "pktDet_autoCorrParams_dout"
  To Register12/1 -> plb_memmap/28  "pktDet_durations_dout"
  To Register11/1 -> plb_memmap/27  "pktDet_thresholds_dout"
  To Register10/1 -> plb_memmap/26  "Tx_Scaling_dout"
  To Register9/1 -> plb_memmap/25  "Tx_Delays_dout"
  To Register8/1 -> plb_memmap/24  "Tx_ControlBits_dout"
  To Register7/1 -> plb_memmap/23  "Tx_Start_Reset_Control_dout"
  To Register6/1 -> plb_memmap/22  "TxRx_FFT_Scaling_dout"
  To Register5/1 -> plb_memmap/21  "TxRx_Interrupt_PktBuf_Ctrl_dout"
  To Register4/1 -> plb_memmap/20  "TxRx_Pilots_Index_dout"
  To Register3/1 -> plb_memmap/19  "TxRx_Pilots_Values_dout"
  To Register2/1 -> plb_memmap/18  "Rx_OFDM_SymbolCounts_dout"
  To Register1/1 -> plb_memmap/17  "Tx_OFDM_SymCounts_dout"
  To Register/1 -> plb_memmap/16  "FEC_Config_dout"
  From Register9/1 -> plb_memmap/15  "pktDet_status_dout"
  From Register8/1 -> plb_memmap/14  "Rx_Gains_dout"
  From Register7/1 -> plb_memmap/13  "Rx_BER_Errors_dout"
  From Register6/1 -> plb_memmap/12  "Rx_BER_TotalBits_dout"
  From Register5/1 -> plb_memmap/11  "Rx_pktDone_interruptStatus_dout"
  From Register4/1 -> plb_memmap/10  "Rx_pilotCFOest_dout"
  From Register3/1 -> plb_memmap/9  "Rx_coarseCFOest_dout"
  From Register2/1 -> plb_memmap/8  "Rx_pktDetEventCount_dout"
  From Register1/1 -> plb_memmap/7  "Tx_PktRunning_dout"
  From Register/1 -> plb_memmap/6  "midPacketRSSI_dout"
  plb_decode/6 -> plb_memmap/4  "RNWReg"
  plb_decode/9 -> plb_memmap/3  "linearAddr"
  plb_decode/5 -> plb_memmap/2  "bankAddr"
  plb_decode/1 -> plb_memmap/1  "wrDBusReg"
  plb_decode/8 -> Sl_rdDBus/1  "Sl_rdDBus"
  plb_decode/7 -> Sl_rdDAck/1  "Sl_rdDAck"
  plb_decode/3 -> Sl_rdComp/1  "Sl_rdComp"
  sg_plb_addrpref/1 -> plb_decode/7  "addrPref"
  PLB_wrDBus/1 -> plb_decode/5  "PLB_wrDBus"
  PLB_RNW/1 -> plb_decode/4  "PLB_RNW"
  PLB_PAValid/1 -> plb_decode/3  "PLB_PAValid"
  PLB_ABus/1 -> plb_decode/2  "PLB_ABus"
  SPLB_Rst/1 -> plb_decode/1  "SPLB_Rst"
  Constant6/1 -> sg_plb_addrpref/1
  Constant5/1 -> Sl_wait/1  "Sl_wait"
  Constant4/1 -> PLB_wrDBus/1
  Constant3/1 -> PLB_RNW/1
  Constant2/1 -> PLB_PAValid/1
  Constant1/1 -> PLB_ABus/1
  Constant/1 -> SPLB_Rst/1
  Sl_wrComp/1 -> Terminator6/1
  Sl_wrDAck/1 -> Terminator5/1
  Sl_wait/1 -> Terminator4/1
  Sl_rdDBus/1 -> Terminator3/1
  Sl_rdDAck/1 -> Terminator2/1
  Sl_rdComp/1 -> Terminator1/1
  Sl_addrAck/1 -> Terminator/1
